simulink model "wlan_phy_rx_pmd"  (Simulink 7.8)
settings: Solver=VariableStepDiscrete  SolverMode=SingleTasking  StartTime=0.0  StopTime=simtime  RelTol=1e-3
PostLoadFcn:
  | wlan_phy_rx_init
InitFcn:
  | wlan_phy_rx_init
StartFcn:
  | wlan_phy_rx_init
values from neighbouring files (symbols this model uses):
  barker_seq20 = 0.95 * circshift(barker_seq20, [0 4]) ./ max(abs(barker_seq20))    [wlan_phy_rx_init.m]
  bit_scrambler_lfsr_bytes = bi2de(reshape(repmat(scr, 1, 8), 8, 127)', 'left-msb')    [wlan_phy_rx_init.m]
  deinterleave_ROM = [deinterleave_ROM interleave_64QAM zeros(1, 512-length(interleave_64QAM))]    [wlan_phy_rx_init.m]
  longCorr_coef_i = [3*real(LTS_corr)]    [wlan_phy_rx_init.m]
  longCorr_coef_q = [3*imag(LTS_corr)]    [wlan_phy_rx_init.m]
  MAX_CP_LEN = 32    [wlan_phy_rx_init.m]
  MAX_NCBPS = 288    [wlan_phy_rx_init.m]
  MAX_NUM_BYTES = 4096    [wlan_phy_rx_init.m]
  MAX_NUM_SC = 64    [wlan_phy_rx_init.m]
  MAX_NUM_SYMS = 600    [wlan_phy_rx_init.m]
  n_bits_preFFT_sampBuff = ceil(log2(4*MAX_NUM_SC))    [wlan_phy_rx_init.m]
  NCBPS_16QAM = 192    [wlan_phy_rx_init.m]
  NCBPS_64QAM = 288    [wlan_phy_rx_init.m]
  NCBPS_BPSK = 48    [wlan_phy_rx_init.m]
  NCBPS_QPSK = 96    [wlan_phy_rx_init.m]
  NDBPS_16QAM12 = 96    [wlan_phy_rx_init.m]
  NDBPS_16QAM34 = 144    [wlan_phy_rx_init.m]
  NDBPS_64QAM23 = 192    [wlan_phy_rx_init.m]
  NDBPS_64QAM34 = 216    [wlan_phy_rx_init.m]
  NDBPS_BPSK12 = 24    [wlan_phy_rx_init.m]
  NDBPS_BPSK34 = 36    [wlan_phy_rx_init.m]
  NDBPS_QPSK12 = 48    [wlan_phy_rx_init.m]
  NDBPS_QPSK34 = 72    [wlan_phy_rx_init.m]
  paylod_vec_samp_time = 8    [wlan_phy_rx_init.m]
  PHY_MIN_PKT_LEN = 14    [wlan_phy_rx_init.m]
  scr_ind_rev = zeros(1,128)    [wlan_phy_rx_init.m]
undefined symbols (not defined in the file or in its folder): gcbh, xlBlockLoadCallback, xlGetNormalizedPeriod, xlipgetpartsetting
libraries: xbsIndex_r4 x3119, simulink x1, xbsEDKLib_r4 x1
(part 4 of 19)

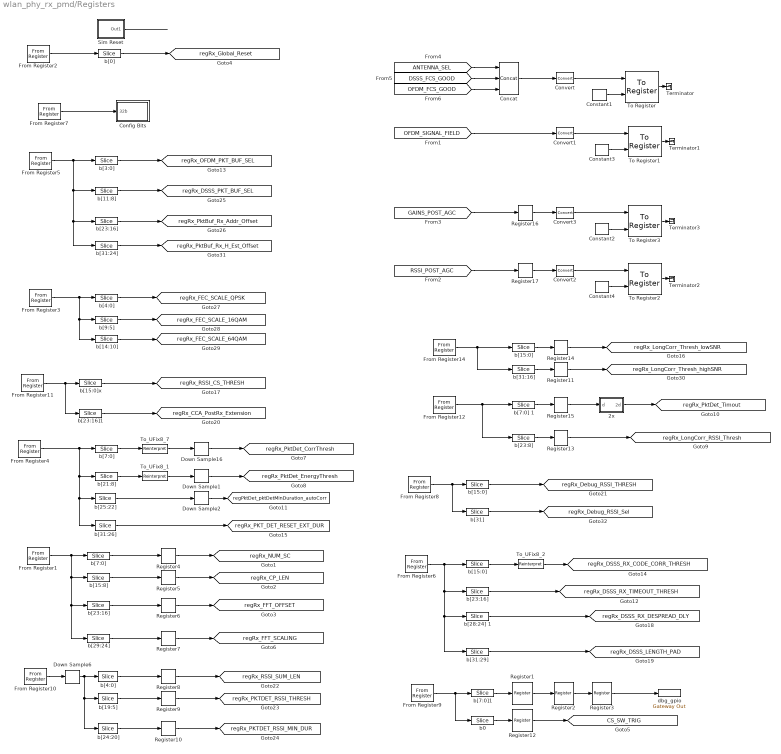
== system wlan_phy_rx_pmd/Registers ==
  " b[23:16] " : xbsIndex_r4/Slice  nbits=8  boolean_output=off  mode=Lower Bit Location + Width  bit1=0  base1=MSB of Input  bit0=16  base0=LSB of Input  dbl_ovrd=off  block_type=slice
      settings: block_version=VER_STRING_GOES_HERE
  " b[31:24]" : xbsIndex_r4/Slice  nbits=8  boolean_output=off  mode=Lower Bit Location + Width  bit1=0  base1=MSB of Input  bit0=24  base0=LSB of Input  dbl_ovrd=off  block_type=slice
      settings: block_version=VER_STRING_GOES_HERE
  "2x " : Subsystem
  Concat : xbsIndex_r4/Concat  num_inputs=3  dbl_ovrd=off  block_type=concat
      settings: block_version=8.2.02
  Config Bits : Subsystem
  Constant{1..4} (4 alike) : xbsIndex_r4/Constant  const=1  gui_display_data_type=Boolean  arith_type=Boolean  n_bits=30  bin_pt=0  preci_type=Single  exp_width=8  frac_width=24  explicit_period=on  period=1  equ=P=C  opselect=C  inp2=PCIN>>17  opr=+  inp1=P  carry=CIN  dbl_ovrd=off  block_type=constant
  Convert{,1..3} (4 alike) : xbsIndex_r4/Convert  gui_display_data_type=Fixed-point  arith_type=Unsigned  n_bits=32  bin_pt=0  float_type=Single  exp_bits=8  fraction_bits=24  quantization=Truncate  overflow=Wrap  en=off  latency=0  dbl_ovrd=off  pipeline=off  block_type=convert
  Down Sample1 : xbsIndex_r4/Down Sample  sample_ratio=8  sample_phase="Last Value of Frame  (most efficient)"  en=off  latency=1  dbl_ovrd=off  block_type=dsamp
      settings: block_version=VER_STRING_GOES_HERE
  Down Sample16 : xbsIndex_r4/Down Sample  sample_ratio=8  sample_phase="Last Value of Frame  (most efficient)"  en=off  latency=1  dbl_ovrd=off  block_type=dsamp
      settings: block_version=VER_STRING_GOES_HERE
  Down Sample2 : xbsIndex_r4/Down Sample  sample_ratio=8  sample_phase="Last Value of Frame  (most efficient)"  en=off  latency=1  dbl_ovrd=off  block_type=dsamp
      settings: block_version=VER_STRING_GOES_HERE
  Down Sample6 : xbsIndex_r4/Down Sample  sample_ratio=16  sample_phase="Last Value of Frame  (most efficient)"  en=off  latency=1  dbl_ovrd=off  block_type=dsamp
      settings: block_version=VER_STRING_GOES_HERE
  From Register1 : xbsIndex_r4/From Register  shared_memory_name='FFT_Config'  init=REG_RX_FFT_Config  period=1  ownership=Locally owned and initialized  gui_display_data_type=Fixed-point  arith_type=Unsigned  n_bits=32  bin_pt=0  preci_type=Single  dbl_ovrd=off  block_type=fromreg
      settings: AttributesFormatString=<< %<shared_memory_name> >>  infoedit="Register block that reads data to a shared memory register.  Delay of one sample period."
  From Register10 : xbsIndex_r4/From Register  shared_memory_name='PKTDET_RSSI_CONFIG'  init=REG_RX_PKTDET_RSSI_CONFIG  period=1  ownership=Locally owned and initialized  gui_display_data_type=Fixed-point  arith_type=Unsigned  n_bits=32  bin_pt=0  preci_type=Single  dbl_ovrd=off  block_type=fromreg
      settings: AttributesFormatString=<< %<shared_memory_name> >>  infoedit="Register block that reads data to a shared memory register.  Delay of one sample period."
  From Register11 : xbsIndex_r4/From Register  shared_memory_name='PHY_CCA_CONFIG'  init=REG_RX_CCA_CONFIG  period=1  ownership=Locally owned and initialized  gui_display_data_type=Fixed-point  arith_type=Unsigned  n_bits=32  bin_pt=0  preci_type=Single  dbl_ovrd=off  block_type=fromreg
      settings: AttributesFormatString=<< %<shared_memory_name> >>  infoedit="Register block that reads data to a shared memory register.  Delay of one sample period."
  From Register12 : xbsIndex_r4/From Register  shared_memory_name='LTS_Corr_Config'  init=REG_RX_LTS_Corr_Confg  period=1  ownership=Locally owned and initialized  gui_display_data_type=Fixed-point  arith_type=Unsigned  n_bits=32  bin_pt=0  preci_type=Single  dbl_ovrd=off  block_type=fromreg
      settings: AttributesFormatString=<< %<shared_memory_name> >>  infoedit="Register block that reads data to a shared memory register.  Delay of one sample period."
  From Register14 : xbsIndex_r4/From Register  shared_memory_name='LTS_Corr_Thresh'  init=REG_RX_LTS_Corr_Thresh  period=1  ownership=Locally owned and initialized  gui_display_data_type=Fixed-point  arith_type=Unsigned  n_bits=32  bin_pt=0  preci_type=Single  dbl_ovrd=off  block_type=fromreg
      settings: AttributesFormatString=<< %<shared_memory_name> >>  infoedit="Register block that reads data to a shared memory register.  Delay of one sample period."
  From Register2 : xbsIndex_r4/From Register  shared_memory_name='Control'  init=REG_RX_Control  period=1  ownership=Locally owned and initialized  gui_display_data_type=Fixed-point  arith_type=Unsigned  n_bits=32  bin_pt=0  preci_type=Single  dbl_ovrd=off  block_type=fromreg
      settings: AttributesFormatString=<< %<shared_memory_name> >>  infoedit="Register block that reads data to a shared memory register.  Delay of one sample period."
  From Register3 : xbsIndex_r4/From Register  shared_memory_name='FEC_CONFIG'  init=REG_RX_FEC_Config  period=1  ownership=Locally owned and initialized  gui_display_data_type=Fixed-point  arith_type=Unsigned  n_bits=32  bin_pt=0  preci_type=Single  dbl_ovrd=off  block_type=fromreg
      settings: AttributesFormatString=<< %<shared_memory_name> >>  infoedit="Register block that reads data to a shared memory register.  Delay of one sample period."
  From Register4 : xbsIndex_r4/From Register  shared_memory_name='PKTDET_AUTOCORR_CONFIG'  init=REG_RX_PktDet_AutoCorr_Config  period=1  ownership=Locally owned and initialized  gui_display_data_type=Fixed-point  arith_type=Unsigned  n_bits=32  bin_pt=0  preci_type=Single  dbl_ovrd=off  block_type=fromreg
      settings: AttributesFormatString=<< %<shared_memory_name> >>  infoedit="Register block that reads data to a shared memory register.  Delay of one sample period."
  From Register5 : xbsIndex_r4/From Register  shared_memory_name='PKT_BUF_SEL'  init=REG_RX_PktBuf_Sel  period=1  ownership=Locally owned and initialized  gui_display_data_type=Fixed-point  arith_type=Unsigned  n_bits=32  bin_pt=0  preci_type=Single  dbl_ovrd=off  block_type=fromreg
      settings: AttributesFormatString=<< %<shared_memory_name> >>  infoedit="Register block that reads data to a shared memory register.  Delay of one sample period."
  From Register6 : xbsIndex_r4/From Register  shared_memory_name='DSSS_RX_CONFIG'  init=REG_RX_DSSS_RX_CONFIG  period=1  ownership=Locally owned and initialized  gui_display_data_type=Fixed-point  arith_type=Unsigned  n_bits=32  bin_pt=0  preci_type=Single  dbl_ovrd=off  block_type=fromreg
      settings: AttributesFormatString=<< %<shared_memory_name> >>  infoedit="Register block that reads data to a shared memory register.  Delay of one sample period."
  From Register7 : xbsIndex_r4/From Register  shared_memory_name='CONFIG'  init=REG_RX_Config  period=1  ownership=Locally owned and initialized  gui_display_data_type=Fixed-point  arith_type=Unsigned  n_bits=32  bin_pt=0  preci_type=Single  dbl_ovrd=off  block_type=fromreg
      settings: AttributesFormatString=<< %<shared_memory_name> >>  infoedit="Register block that reads data to a shared memory register.  Delay of one sample period."
  From Register8 : xbsIndex_r4/From Register  shared_memory_name='RSSI_THRESH'  init=600  period=1  ownership=Locally owned and initialized  gui_display_data_type=Fixed-point  arith_type=Unsigned  n_bits=32  bin_pt=0  preci_type=Single  dbl_ovrd=off  block_type=fromreg
      settings: AttributesFormatString=<< %<shared_memory_name> >>  infoedit="Register block that reads data to a shared memory register.  Delay of one sample period."
  From Register9 : xbsIndex_r4/From Register  shared_memory_name='DEBUG_GPIO'  init=0  period=1  ownership=Locally owned and initialized  gui_display_data_type=Fixed-point  arith_type=Unsigned  n_bits=32  bin_pt=0  preci_type=Single  dbl_ovrd=off  block_type=fromreg
      settings: AttributesFormatString=<< %<shared_memory_name> >>  infoedit="Register block that reads data to a shared memory register.  Delay of one sample period."
  Register{1..17} (17 alike) : xbsIndex_r4/Register  init=0  rst=off  en=off  dbl_ovrd=off  block_type=register
  Sim Reset : Subsystem
  Terminator{,1..3} (4 alike) : Terminator
  To Register : xbsIndex_r4/To Register  shared_memory_name='Status'  init=0  ownership=Locally owned and initialized  explicit_data_type=on  gui_display_data_type=Fixed-point  arith_type=Unsigned  n_bits=32  bin_pt=0  preci_type=Single  dbl_ovrd=off  block_type=toreg
      settings: AttributesFormatString=<< %<shared_memory_name> >>  infoedit="Register block that writes data to a shared memory register.  Delay of one sample period."
  To Register1 : xbsIndex_r4/To Register  shared_memory_name='SIGNAL_FIELD'  init=0  ownership=Locally owned and initialized  explicit_data_type=on  gui_display_data_type=Fixed-point  arith_type=Unsigned  n_bits=32  bin_pt=0  preci_type=Single  dbl_ovrd=off  block_type=toreg
      settings: AttributesFormatString=<< %<shared_memory_name> >>  infoedit="Register block that writes data to a shared memory register.  Delay of one sample period."
  To Register2 : xbsIndex_r4/To Register  shared_memory_name='RX_PKT_RSSI_AB'  init=0  ownership=Locally owned and initialized  explicit_data_type=on  gui_display_data_type=Fixed-point  arith_type=Unsigned  n_bits=32  bin_pt=0  preci_type=Single  dbl_ovrd=off  block_type=toreg
      settings: AttributesFormatString=<< %<shared_memory_name> >>  infoedit="Register block that writes data to a shared memory register.  Delay of one sample period."
  To Register3 : xbsIndex_r4/To Register  shared_memory_name='RX_PKT_AGC_GAINS'  init=0  ownership=Locally owned and initialized  explicit_data_type=on  gui_display_data_type=Fixed-point  arith_type=Unsigned  n_bits=32  bin_pt=0  preci_type=Single  dbl_ovrd=off  block_type=toreg
      settings: AttributesFormatString=<< %<shared_memory_name> >>  infoedit="Register block that writes data to a shared memory register.  Delay of one sample period."
  To_UFix8_1 : xbsIndex_r4/Reinterpret  force_arith_type=on  arith_type=Unsigned  force_bin_pt=on  bin_pt=8  block_type=cast
      settings: block_version=VER_STRING_GOES_HERE
  To_UFix8_2 : xbsIndex_r4/Reinterpret  force_arith_type=on  arith_type=Unsigned  force_bin_pt=on  bin_pt=8  block_type=cast
      settings: block_version=VER_STRING_GOES_HERE
  To_UFix8_7 : xbsIndex_r4/Reinterpret  force_arith_type=on  arith_type=Unsigned  force_bin_pt=on  bin_pt=8  block_type=cast
      settings: block_version=VER_STRING_GOES_HERE
  b0 : xbsIndex_r4/Slice  nbits=1  boolean_output=off  mode=Lower Bit Location + Width  bit1=0  base1=MSB of Input  bit0=0  base0=LSB of Input  dbl_ovrd=off  block_type=slice
      settings: block_version=VER_STRING_GOES_HERE
  b[0] : xbsIndex_r4/Slice  nbits=1  boolean_output=off  mode=Lower Bit Location + Width  bit1=0  base1=MSB of Input  bit0=0  base0=LSB of Input  dbl_ovrd=off  block_type=slice
      settings: block_version=VER_STRING_GOES_HERE
  b[11:8] : xbsIndex_r4/Slice  nbits=4  boolean_output=off  mode=Lower Bit Location + Width  bit1=0  base1=MSB of Input  bit0=8  base0=LSB of Input  dbl_ovrd=off  block_type=slice
      settings: block_version=VER_STRING_GOES_HERE
  b[14:10] : xbsIndex_r4/Slice  nbits=5  boolean_output=off  mode=Lower Bit Location + Width  bit1=0  base1=MSB of Input  bit0=10  base0=LSB of Input  dbl_ovrd=off  block_type=slice
      settings: block_version=VER_STRING_GOES_HERE
  b[15:0] : xbsIndex_r4/Slice  nbits=16  boolean_output=off  mode=Lower Bit Location + Width  bit1=0  base1=MSB of Input  bit0=0  base0=LSB of Input  dbl_ovrd=off  block_type=slice
      settings: block_version=VER_STRING_GOES_HERE
  "b[15:0]   " : xbsIndex_r4/Slice  nbits=16  boolean_output=off  mode=Lower Bit Location + Width  bit1=0  base1=MSB of Input  bit0=0  base0=LSB of Input  dbl_ovrd=off  block_type=slice
      settings: block_version=VER_STRING_GOES_HERE
  "b[15:0]    " : xbsIndex_r4/Slice  nbits=16  boolean_output=off  mode=Lower Bit Location + Width  bit1=0  base1=MSB of Input  bit0=0  base0=LSB of Input  dbl_ovrd=off  block_type=slice
      settings: block_version=VER_STRING_GOES_HERE
  b[15:0]x : xbsIndex_r4/Slice  nbits=16  boolean_output=off  mode=Lower Bit Location + Width  bit1=0  base1=MSB of Input  bit0=0  base0=LSB of Input  dbl_ovrd=off  block_type=slice
      settings: block_version=VER_STRING_GOES_HERE
  b[15:8] : xbsIndex_r4/Slice  nbits=8  boolean_output=off  mode=Lower Bit Location + Width  bit1=0  base1=MSB of Input  bit0=8  base0=LSB of Input  dbl_ovrd=off  block_type=slice
      settings: block_version=VER_STRING_GOES_HERE
  b[19:5] : xbsIndex_r4/Slice  nbits=15  boolean_output=off  mode=Lower Bit Location + Width  bit1=0  base1=MSB of Input  bit0=5  base0=LSB of Input  dbl_ovrd=off  block_type=slice
      settings: block_version=VER_STRING_GOES_HERE
  "b[21:8] " : xbsIndex_r4/Slice  nbits=14  boolean_output=off  mode=Lower Bit Location + Width  bit1=0  base1=MSB of Input  bit0=8  base0=LSB of Input  dbl_ovrd=off  block_type=slice
      settings: block_version=VER_STRING_GOES_HERE
  b[23:16] : xbsIndex_r4/Slice  nbits=8  boolean_output=off  mode=Lower Bit Location + Width  bit1=0  base1=MSB of Input  bit0=16  base0=LSB of Input  dbl_ovrd=off  block_type=slice
      settings: block_version=VER_STRING_GOES_HERE
  "b[23:16]   " : xbsIndex_r4/Slice  nbits=8  boolean_output=off  mode=Lower Bit Location + Width  bit1=0  base1=MSB of Input  bit0=16  base0=LSB of Input  dbl_ovrd=off  block_type=slice
      settings: block_version=VER_STRING_GOES_HERE
  b[23:16]1 : xbsIndex_r4/Slice  nbits=8  boolean_output=off  mode=Lower Bit Location + Width  bit1=0  base1=MSB of Input  bit0=16  base0=LSB of Input  dbl_ovrd=off  block_type=slice
      settings: block_version=VER_STRING_GOES_HERE
  b[23:8] : xbsIndex_r4/Slice  nbits=16  boolean_output=off  mode=Lower Bit Location + Width  bit1=0  base1=MSB of Input  bit0=8  base0=LSB of Input  dbl_ovrd=off  block_type=slice
      settings: block_version=VER_STRING_GOES_HERE
  b[24:20] : xbsIndex_r4/Slice  nbits=5  boolean_output=off  mode=Lower Bit Location + Width  bit1=0  base1=MSB of Input  bit0=20  base0=LSB of Input  dbl_ovrd=off  block_type=slice
      settings: block_version=VER_STRING_GOES_HERE
  "b[25:22] " : xbsIndex_r4/Slice  nbits=4  boolean_output=off  mode=Lower Bit Location + Width  bit1=0  base1=MSB of Input  bit0=22  base0=LSB of Input  dbl_ovrd=off  block_type=slice
      settings: block_version=VER_STRING_GOES_HERE
  b[28:24] 1 : xbsIndex_r4/Slice  nbits=5  boolean_output=off  mode=Lower Bit Location + Width  bit1=0  base1=MSB of Input  bit0=24  base0=LSB of Input  dbl_ovrd=off  block_type=slice
      settings: block_version=VER_STRING_GOES_HERE
  b[29:24] : xbsIndex_r4/Slice  nbits=6  boolean_output=off  mode=Lower Bit Location + Width  bit1=0  base1=MSB of Input  bit0=24  base0=LSB of Input  dbl_ovrd=off  block_type=slice
      settings: block_version=VER_STRING_GOES_HERE
  b[31:16] : xbsIndex_r4/Slice  nbits=16  boolean_output=off  mode=Lower Bit Location + Width  bit1=0  base1=MSB of Input  bit0=16  base0=LSB of Input  dbl_ovrd=off  block_type=slice
      settings: block_version=VER_STRING_GOES_HERE
  b[31:26] : xbsIndex_r4/Slice  nbits=6  boolean_output=off  mode=Lower Bit Location + Width  bit1=0  base1=MSB of Input  bit0=26  base0=LSB of Input  dbl_ovrd=off  block_type=slice
      settings: block_version=VER_STRING_GOES_HERE
  b[31:29] : xbsIndex_r4/Slice  nbits=3  boolean_output=off  mode=Lower Bit Location + Width  bit1=0  base1=MSB of Input  bit0=29  base0=LSB of Input  dbl_ovrd=off  block_type=slice
      settings: block_version=VER_STRING_GOES_HERE
  b[31] : xbsIndex_r4/Slice  nbits=1  boolean_output=off  mode=Lower Bit Location + Width  bit1=0  base1=MSB of Input  bit0=31  base0=LSB of Input  dbl_ovrd=off  block_type=slice
      settings: block_version=VER_STRING_GOES_HERE
  b[3:0] : xbsIndex_r4/Slice  nbits=4  boolean_output=off  mode=Lower Bit Location + Width  bit1=0  base1=MSB of Input  bit0=0  base0=LSB of Input  dbl_ovrd=off  block_type=slice
      settings: block_version=VER_STRING_GOES_HERE
  b[4:0] : xbsIndex_r4/Slice  nbits=5  boolean_output=off  mode=Lower Bit Location + Width  bit1=0  base1=MSB of Input  bit0=0  base0=LSB of Input  dbl_ovrd=off  block_type=slice
      settings: block_version=VER_STRING_GOES_HERE
  "b[4:0]  " : xbsIndex_r4/Slice  nbits=5  boolean_output=off  mode=Lower Bit Location + Width  bit1=0  base1=MSB of Input  bit0=0  base0=LSB of Input  dbl_ovrd=off  block_type=slice
      settings: block_version=VER_STRING_GOES_HERE
  b[7:0] : xbsIndex_r4/Slice  nbits=8  boolean_output=off  mode=Lower Bit Location + Width  bit1=0  base1=MSB of Input  bit0=0  base0=LSB of Input  dbl_ovrd=off  block_type=slice
      settings: block_version=VER_STRING_GOES_HERE
  "b[7:0] " : xbsIndex_r4/Slice  nbits=8  boolean_output=off  mode=Lower Bit Location + Width  bit1=0  base1=MSB of Input  bit0=0  base0=LSB of Input  dbl_ovrd=off  block_type=slice
      settings: block_version=VER_STRING_GOES_HERE
  b[7:0] 1 : xbsIndex_r4/Slice  nbits=8  boolean_output=off  mode=Lower Bit Location + Width  bit1=0  base1=MSB of Input  bit0=0  base0=LSB of Input  dbl_ovrd=off  block_type=slice
      settings: block_version=VER_STRING_GOES_HERE
  b[7:0]1 : xbsIndex_r4/Slice  nbits=8  boolean_output=off  mode=Lower Bit Location + Width  bit1=0  base1=MSB of Input  bit0=0  base0=LSB of Input  dbl_ovrd=off  block_type=slice
      settings: block_version=VER_STRING_GOES_HERE
  b[9:5] : xbsIndex_r4/Slice  nbits=5  boolean_output=off  mode=Lower Bit Location + Width  bit1=0  base1=MSB of Input  bit0=5  base0=LSB of Input  dbl_ovrd=off  block_type=slice
      settings: block_version=VER_STRING_GOES_HERE
  dbg_gpio : xbsIndex_r4/Gateway Out  inherit_from_input=off  hdl_port=on  timing_constraint=None  locs_specified=off  LOCs={}  UseAsDAC=off  DACChannel='1'  block_type=gatewayout
  tag scope: CS_SW_TRIG=global, regPktDet_pktDetMinDuration_autoCorr=global, regRx_CCA_PostRx_Extension=global, regRx_CP_LEN=global, regRx_DSSS_LENGTH_PAD=global, regRx_DSSS_PKT_BUF_SEL=global, regRx_DSSS_RX_CODE_CORR_THRESH=global, regRx_DSSS_RX_DESPREAD_DLY=global, regRx_DSSS_RX_TIMEOUT_THRESH=global, regRx_Debug_RSSI_Sel=global, regRx_Debug_RSSI_THRESH=global, regRx_FEC_SCALE_16QAM=global, regRx_FEC_SCALE_64QAM=global, regRx_FEC_SCALE_QPSK=global, regRx_FFT_OFFSET=global, regRx_FFT_SCALING=global, regRx_Global_Reset=global, regRx_LongCorr_RSSI_Thresh=global, regRx_LongCorr_Thresh_highSNR=global, regRx_LongCorr_Thresh_lowSNR=global, regRx_NUM_SC=global, regRx_OFDM_PKT_BUF_SEL=global, regRx_PKTDET_RSSI_MIN_DUR=global, regRx_PKTDET_RSSI_THRESH=global, regRx_PKT_DET_RESET_EXT_DUR=global, regRx_PktBuf_Rx_Addr_Offset=global, regRx_PktBuf_Rx_H_Est_Offset=global, regRx_PktDet_CorrThresh=global, regRx_PktDet_EnergyThresh=global, regRx_PktDet_Timout=global, regRx_RSSI_CS_THRESH=global, regRx_RSSI_SUM_LEN=global
  wires:
  b[31:16]/1 -> Register11/1
  From Register1/1 -> b[7:0]/1, b[15:8]/1, b[23:16]/1, b[29:24]/1
  b[7:0]/1 -> Register4/1
  b[15:8]/1 -> Register5/1
  b[23:16]/1 -> Register6/1
  From Register2/1 -> b[0]/1
  b[0]/1 -> [regRx_Global_Reset]
  From Register3/1 -> "b[4:0]  "/1, b[9:5]/1, b[14:10]/1
  b[29:24]/1 -> Register7/1
  [DSSS_FCS_GOOD] -> Concat/2
  [OFDM_FCS_GOOD] -> Concat/3
  Constant1/1 -> To Register/2
  To Register/1 -> Terminator/1
  From Register4/1 -> "b[7:0] "/1, "b[21:8] "/1, "b[25:22] "/1, b[31:26]/1
  "b[7:0] "/1 -> To_UFix8_7/1
  "b[21:8] "/1 -> To_UFix8_1/1
  To_UFix8_7/1 -> Down Sample16/1
  To_UFix8_1/1 -> Down Sample1/1
  Down Sample16/1 -> [regRx_PktDet_CorrThresh]
  Down Sample1/1 -> [regRx_PktDet_EnergyThresh]
  b[15:0]/1 -> Register14/1
  "b[25:22] "/1 -> Down Sample2/1
  Down Sample2/1 -> [regPktDet_pktDetMinDuration_autoCorr]
  Sim Reset/1(Out1) -> ?
  From Register5/1 -> b[3:0]/1, b[11:8]/1, " b[23:16] "/1, " b[31:24]"/1
  b[3:0]/1 -> [regRx_OFDM_PKT_BUF_SEL]
  From Register6/1 -> "b[15:0]   "/1, "b[23:16]   "/1, b[28:24] 1/1, b[31:29]/1
  "b[15:0]   "/1 -> To_UFix8_2/1
  "b[23:16]   "/1 -> [regRx_DSSS_RX_TIMEOUT_THRESH]
  To_UFix8_2/1 -> [regRx_DSSS_RX_CODE_CORR_THRESH]
  Concat/1 -> Convert/1
  b[28:24] 1/1 -> [regRx_DSSS_RX_DESPREAD_DLY]
  b[31:29]/1 -> [regRx_DSSS_LENGTH_PAD]
  From Register8/1 -> "b[15:0]    "/1, b[31]/1
  "b[15:0]    "/1 -> [regRx_Debug_RSSI_THRESH]
  From Register9/1 -> b[7:0]1/1, b0/1
  b[7:0]1/1 -> Register1/1
  Register1/1 -> Register2/1
  Register2/1 -> Register3/1
  Register3/1 -> dbg_gpio/1
  b[4:0]/1 -> Register8/1
  Down Sample6/1 -> b[4:0]/1, b[19:5]/1, b[24:20]/1
  b[19:5]/1 -> Register9/1
  From Register10/1 -> Down Sample6/1
  b[24:20]/1 -> Register10/1
  From Register7/1 -> Config Bits/1(32b)
  Register4/1 -> [regRx_NUM_SC]
  Register5/1 -> [regRx_CP_LEN]
  Register6/1 -> [regRx_FFT_OFFSET]
  Register7/1 -> [regRx_FFT_SCALING]
  Register8/1 -> [regRx_RSSI_SUM_LEN]
  Register9/1 -> [regRx_PKTDET_RSSI_THRESH]
  Register10/1 -> [regRx_PKTDET_RSSI_MIN_DUR]
  Register11/1 -> [regRx_LongCorr_Thresh_highSNR]
  b[31:26]/1 -> [regRx_PKT_DET_RESET_EXT_DUR]
  From Register11/1 -> b[15:0]x/1, b[23:16]1/1
  b[15:0]x/1 -> [regRx_RSSI_CS_THRESH]
  b[23:16]1/1 -> [regRx_CCA_PostRx_Extension]
  b[11:8]/1 -> [regRx_DSSS_PKT_BUF_SEL]
  Convert/1 -> To Register/1
  " b[23:16] "/1 -> [regRx_PktBuf_Rx_Addr_Offset]
  "b[4:0]  "/1 -> [regRx_FEC_SCALE_QPSK]
  b[9:5]/1 -> [regRx_FEC_SCALE_16QAM]
  b[14:10]/1 -> [regRx_FEC_SCALE_64QAM]
  Constant3/1 -> To Register1/2
  To Register1/1 -> Terminator1/1
  [OFDM_SIGNAL_FIELD] -> Convert1/1
  Convert1/1 -> To Register1/1
  b0/1 -> Register12/1
  Register12/1 -> [CS_SW_TRIG]
  Constant4/1 -> To Register2/2
  To Register2/1 -> Terminator2/1
  Convert2/1 -> To Register2/1
  Register13/1 -> [regRx_LongCorr_RSSI_Thresh]
  b[23:8]/1 -> Register13/1
  Register14/1 -> [regRx_LongCorr_Thresh_lowSNR]
  From Register14/1 -> b[15:0]/1, b[31:16]/1
  From Register12/1 -> b[7:0] 1/1, b[23:8]/1
  "2x "/1(2d) -> [regRx_PktDet_Timout]
  Register15/1 -> "2x "/1(d)
  b[7:0] 1/1 -> Register15/1
  " b[31:24]"/1 -> [regRx_PktBuf_Rx_H_Est_Offset]
  [RSSI_POST_AGC] -> Register17/1
  [ANTENNA_SEL] -> Concat/1
  Constant2/1 -> To Register3/2
  To Register3/1 -> Terminator3/1
  Convert3/1 -> To Register3/1
  [GAINS_POST_AGC] -> Register16/1
  Register16/1 -> Convert3/1
  Register17/1 -> Convert2/1
  b[31]/1 -> [regRx_Debug_RSSI_Sel]

== system wlan_phy_rx_pmd/Resets ==
  Convert1 : xbsIndex_r4/Convert  gui_display_data_type=Boolean  arith_type=Boolean  n_bits=16  bin_pt=14  float_type=Single  exp_bits=8  fraction_bits=24  quantization=Truncate  overflow=Wrap  en=off  latency=0  dbl_ovrd=off  pipeline=off  block_type=convert
  Pulse Generator : DiscretePulseGenerator  Period=1e9  PulseWidth=8  PhaseDelay=100
  tag scope: RX_GLOBAL_RESET=global
  wires:
  [regRx_Global_Reset] -> Convert1/1
  Convert1/1 -> [RX_GLOBAL_RESET]

== system wlan_phy_rx_pmd/Sync & Antenna Sel ==
  Rx I : Inport
  Rx Q : Inport  Port=2
  IQ Valid : Inport  Port=3
  RSSI : Inport  Port=4
  Reset : Inport  Port=5
  ADC/Corr : Scope  NumInputPorts=10
      settings: TimeRange=16708  YMin=0~-1~-1~0~-1~-1~-1~-1~-1~-1  YMax=1~1~1~1~1~1~1~1~1~1  SaveName=ScopeData34
  Antenna Selection : Subsystem
  Debug  Outputs : Subsystem
  From Workspace1 : FromWorkspace  VariableName=samps_pkt_det  SampleTime=paylod_vec_samp_time  Interpolate=off  OutputAfterFinalValue=Setting to zero
  Gateway Out{,1..3} (4 alike) : xbsIndex_r4/Gateway Out  inherit_from_input=off  hdl_port=off  timing_constraint=None  locs_specified=off  LOCs={}  UseAsDAC=off  DACChannel='1'  block_type=gatewayout
  LTS Correlation : Subsystem
  PHY CCA : Subsystem
  Pkt Det : Subsystem
  Pkt Det AGC Ctrl : Subsystem
  Resets : Subsystem
  " Rx I" : Outport
  " Rx Q" : Outport  Port=2
  " IQ Valid" : Outport  Port=3
  " Pkt Det" : Outport  Port=4
  LTS Sync : Outport  Port=5
  wires:
  LTS Correlation/1(Det) -> LTS Sync/1, PHY CCA/1(LTS Sync), Debug  Outputs/2(LTS)
  LTS Correlation/2(Timeout) -> PHY CCA/2(LTS Timeout), Resets/3(LTS Timeout), Debug  Outputs/3(LTS Timeout), Gateway Out2/1
  From Workspace1/1 -> ADC/Corr/10  "Capt Pkt Det"
  Gateway Out2/1 -> ADC/Corr/3  "LTS TO"
  Gateway Out1/1 -> ADC/Corr/2  "Pkt Done"
  Gateway Out/1 -> ADC/Corr/1  "Pkt Det"
  Pkt Det/1(Pkt Det) -> Antenna Selection/1(Pkt Det), LTS Correlation/5(En), PHY CCA/4(Pkt Det), Debug  Outputs/1(Pkt Det), " Pkt Det"/1, Pkt Det AGC Ctrl/1(Pkt Det), Resets/1(Det), Gateway Out/1
  Resets/1(Reset) -> Pkt Det/1(Reset), Antenna Selection/6(Reset), LTS Correlation/6(Reset), PHY CCA/6(Reset), Gateway Out1/1
  Reset/1 -> Resets/2(OFDM Reset)
  IQ Valid/1 -> Pkt Det/4(IQ Valid), Antenna Selection/4(IQ Valid)
  RSSI/1 -> Pkt Det/5(RSSI Vec), Antenna Selection/5(RSSI Vec), PHY CCA/5(Avg RSSI Vec)
  Rx I/1 -> Pkt Det/2(Rx I Vec), Antenna Selection/2(Rx I Vec)
  Rx Q/1 -> Pkt Det/3(Rx Q Vec), Antenna Selection/3(Rx Q Vec)
  Antenna Selection/1(Rx I) -> " Rx I"/1, LTS Correlation/1(rx_I)
  Antenna Selection/2(" Rx Q") -> " Rx Q"/1, LTS Correlation/2(rx_Q)
  Antenna Selection/3(" IQ Valid") -> " IQ Valid"/1, LTS Correlation/3(rx_IQ_valid), PHY CCA/3(IQ Valid)
  Antenna Selection/4(RSSI) -> LTS Correlation/4(RSSI), Gateway Out3/1  "Avg RSSI"
  Gateway Out3/1 -> ADC/Corr/9

== system wlan_phy_rx_pmd/CFO & Samp Buffer/CFO Correction ==
  note: DDS requires >1 cycle EN early to get setup
      | Use gloabl reset for this
      | Safe, since DDS output is ignored when
      | it's not being used, so bogus outputs
      | between packets is no problem
      |
      | DDS updates once per input sample
      | Since the FFT only consumes 64 samples
      | per OFDM symbol (ignoring the CP),
      | the DDS keeps running after each FFT
      | frame is loaded. This is fine, since the FFT is
      | configured for non-streaming (it ignores its
      | I/Q inputs while computing a transform)
  "I " : Inport
  "Q " : Inport  Port=2
  DDS Run : Inport  Port=3
  Valid : Inport  Port=4
  tlast : Inport  Port=5
  CFO Est : Inport  Port=6
  CFO Corr : Scope  NumInputPorts=8
      settings: TimeRange=35000  YMin=0~-0.4~0~-1~0~0~-0.4~0  YMax=1~0.4~1~2~1~1~0.4~1  SaveName=ScopeData3
  "Complex Multiplier 5.0 " : xbsIndex_r4/Complex Multiplier 5.0   hasatlast=off  hasatuser=on  atuserwidth=1  hasbtlast=off  hasbtuser=off  btuserwidth=1  multtype=Use_Mults  optimizegoal=Performance  flowcontrol=NonBlocking  outputwidth=16  roundmode=Truncate  hasctrltlast=off  hasctrltuser=off  ctrltuserwidth=1  outtlastbehv=Null  latencyconfig=Manual  minimumlatency=2  aclken=off  aresetn=off  trim_axipin_name=on  aportwidth=63  bportwidth=63  ip_name=Complex Multiplier  ip_version=5.0  dsptool_ready=true  ipcore_usecache=true  ipcore_useipmodelcache=true  ipcore_verbose=false  ipcore_latency_parameter='minimumlatency'  wrapper_available=true  port_translation_map={ 'aclken' => 'en', 'aresetn' => 'rst'}  structural_sim=false  block_type=cmpy_v5_0
  Constant1 : xbsIndex_r4/Constant  const=1  gui_display_data_type=Boolean  arith_type=Boolean  n_bits=16  bin_pt=14  preci_type=Single  exp_width=8  frac_width=24  explicit_period=off  period=8  equ=P=C  opselect=C  inp2=PCIN>>17  opr=+  inp1=P  carry=CIN  dbl_ovrd=off  block_type=constant
  Constant2 : xbsIndex_r4/Constant  const=1  gui_display_data_type=Fixed-point  arith_type=Signed (2's comp)  n_bits=16  bin_pt=15  preci_type=Single  exp_width=8  frac_width=24  explicit_period=on  period=1  equ=P=C  opselect=C  inp2=PCIN>>17  opr=+  inp1=P  carry=CIN  dbl_ovrd=off  block_type=constant
  Constant3 : xbsIndex_r4/Constant  const=0  gui_display_data_type=Fixed-point  arith_type=Signed (2's comp)  n_bits=16  bin_pt=15  preci_type=Single  exp_width=8  frac_width=24  explicit_period=on  period=1  equ=P=C  opselect=C  inp2=PCIN>>17  opr=+  inp1=P  carry=CIN  dbl_ovrd=off  block_type=constant
  Constant5 : xbsIndex_r4/Constant  const=1  gui_display_data_type=Boolean  arith_type=Boolean  n_bits=16  bin_pt=14  preci_type=Single  exp_width=8  frac_width=24  explicit_period=off  period=8  equ=P=C  opselect=C  inp2=PCIN>>17  opr=+  inp1=P  carry=CIN  dbl_ovrd=off  block_type=constant
  Convert1 : xbsIndex_r4/Convert  gui_display_data_type=Fixed-point  arith_type="Signed  (2's comp)"  n_bits=16  bin_pt=15  float_type=Single  exp_bits=8  fraction_bits=24  quantization=Truncate  overflow=Wrap  en=off  latency=0  dbl_ovrd=off  pipeline=off  block_type=convert
  Convert2 : xbsIndex_r4/Convert  gui_display_data_type=Fixed-point  arith_type="Signed  (2's comp)"  n_bits=16  bin_pt=15  float_type=Single  exp_bits=8  fraction_bits=24  quantization=Truncate  overflow=Wrap  en=off  latency=0  dbl_ovrd=off  pipeline=off  block_type=convert
  Convert3 : xbsIndex_r4/Convert  gui_display_data_type=Fixed-point  arith_type="Signed  (2's comp)"  n_bits=26  bin_pt=26  float_type=Single  exp_bits=8  fraction_bits=24  quantization=Truncate  overflow=Flag as error  en=off  latency=0  dbl_ovrd=off  pipeline=off  block_type=convert
  Convert4 : xbsIndex_r4/Convert  gui_display_data_type=Fixed-point  arith_type=Unsigned  n_bits=1  bin_pt=0  float_type=Single  exp_bits=8  fraction_bits=24  quantization=Truncate  overflow=Flag as error  en=off  latency=0  dbl_ovrd=off  pipeline=off  block_type=convert
  Convert5 : xbsIndex_r4/Convert  gui_display_data_type=Boolean  arith_type=Boolean  n_bits=1  bin_pt=0  float_type=Single  exp_bits=8  fraction_bits=24  quantization=Truncate  overflow=Flag as error  en=off  latency=0  dbl_ovrd=off  pipeline=off  block_type=convert
  DDS Compiler 4.0 : xbsIndex_r4/DDS Compiler 4.0   partspresent=Phase_Generator_and_SIN_COS_LUT  dds_clock_rate=160  channels=1  parameter_entry=Hardware_Parameters  spurious_free_dynamic_range=36  frequency_resolution=0.4  noise_shaping=None  phase_width=26  output_width=16  output_selection=Sine_and_Cosine  negative_sine=off  negative_cosine=off  amplitude_mode=Full_Range  memory_type=Auto  optimization_goal=Auto  dsp48_use=Minimal  latency_configuration=Auto  latency=6  has_phase_out=off  sclr_pin=off  clock_enable=on  rfd=off  rdy=on  channel_pin=off  explicit_period=off  period=1  phase_increment=Programmable  output_frequency1=0  output_frequency2=0  output_frequency3=0  output_frequency4=0  output_frequency5=0  output_frequency6=0  output_frequency7=0  output_frequency8=0  output_frequency9=0  output_frequency10=0  output_frequency11=0  output_frequency12=0  output_frequency13=0  output_frequency14=0  output_frequency15=0  output_frequency16=0  pinc1='0'  pinc2='0'  pinc3='0'  pinc4='0'  pinc5='0'  pinc6='0'  pinc7='0'  pinc8='0'  pinc9='0'  pinc10='0'  pinc11='0'  pinc12='0'  pinc13='0'  pinc14='0'  pinc15='0'  pinc16='0'  phase_offset=None  phase_offset_angles1=0  phase_offset_angles2=0  phase_offset_angles3=0  phase_offset_angles4=0  phase_offset_angles5=0  phase_offset_angles6=0  phase_offset_angles7=0  phase_offset_angles8=0  phase_offset_angles9=0  phase_offset_angles10=0  phase_offset_angles11=0  phase_offset_angles12=0  phase_offset_angles13=0  phase_offset_angles14=0  phase_offset_angles15=0  phase_offset_angles16=0  poff1='0'  poff2='0'  poff3='0'  poff4='0'  poff5='0'  poff6='0'  poff7='0'  poff8='0'  poff9='0'  poff10='0'  poff11='0'  poff12='0'  poff13='0'  poff14='0'  poff15='0'  poff16='0'  por_mode=false  gui_behaviour=Sysgen  ip_name=DDS Compiler  ip_version=4.0  dsptool_ready=true  wrapper_available=true  port_translation_map={ 'ce' => 'en', 'sclr' => 'rst' }  ipcore_xco_need_fpga_part=true  ipcore_fpga_part=xlipgetpartsetting(gcb, {'virtex4', 'xc4vsx35', '-10', 'ff668'})  ipcore_usecache=true  ipcore_useipmodelcache=true  ipcore_verbose=false  block_type=dds_compiler_v4_0
  Gateway Out{1..7,17} (8 alike) : xbsIndex_r4/Gateway Out  inherit_from_input=off  hdl_port=off  timing_constraint=None  locs_specified=off  LOCs={}  UseAsDAC=off  DACChannel='1'  block_type=gatewayout
  Logical2 : xbsIndex_r4/Logical  logical_function=OR  inputs=3  en=off  latency=0  precision=Full  arith_type=Unsigned  n_bits=16  bin_pt=0  align_bp=on  dbl_ovrd=off  block_type=logical
  Sim Reset : Subsystem
  valid : Outport
  I : Outport  Port=2
  Q : Outport  Port=3
  " tlast" : Outport  Port=4
  wires:
  Constant1/1 -> DDS Compiler 4.0/1
  CFO Est/1 -> Convert3/1
  DDS Run/1 -> Logical2/1
  "Complex Multiplier 5.0 "/2 -> Convert2/1, Gateway Out6/1
  "Complex Multiplier 5.0 "/3 -> Convert1/1
  "I "/1 -> "Complex Multiplier 5.0 "/3, Gateway Out1/1
  "Q "/1 -> "Complex Multiplier 5.0 "/2
  Gateway Out17/1 -> CFO Corr/1
  Gateway Out1/1 -> CFO Corr/2
  Gateway Out2/1 -> CFO Corr/3
  Gateway Out3/1 -> CFO Corr/4
  Gateway Out4/1 -> CFO Corr/5
  DDS Compiler 4.0/2 -> "Complex Multiplier 5.0 "/6, Gateway Out3/1
  Valid/1 -> "Complex Multiplier 5.0 "/1, Gateway Out2/1
  Convert1/1 -> I/1
  Convert2/1 -> Q/1
  "Complex Multiplier 5.0 "/1 -> valid/1, Gateway Out5/1
  DDS Compiler 4.0/3 -> "Complex Multiplier 5.0 "/7
  Logical2/1 -> DDS Compiler 4.0/3, Gateway Out17/1
  [RX_GLOBAL_RESET] -> Logical2/2
  Convert3/1 -> DDS Compiler 4.0/2
  Constant5/1 -> "Complex Multiplier 5.0 "/5
  tlast/1 -> Convert4/1
  "Complex Multiplier 5.0 "/4 -> Gateway Out7/1, Convert5/1
  Gateway Out5/1 -> CFO Corr/6
  Gateway Out6/1 -> CFO Corr/7
  Gateway Out7/1 -> CFO Corr/8
  Convert4/1 -> "Complex Multiplier 5.0 "/4, Gateway Out4/1
  Convert5/1 -> " tlast"/1
  Sim Reset/1(Out1) -> Logical2/3

== system wlan_phy_rx_pmd/CFO & Samp Buffer/CFO Estimation ==
  note: (1/2) for normalization of phase
      | (1/64) for number of summed elements
  LTS_Sync : Inport
  rx_I : Inport  Port=2
  rx_Q : Inport  Port=3
  rx_valid : Inport  Port=4
  CFO Est : Scope  NumInputPorts=10
      settings: TimeRange=10000  YMin=-0.25~-0.25~-0.04~-0.05~-0.3~-0.125~-1~0~-0.000175~-1  YMax=0.3~0.3~0.05~0.04~0.9~0.15~1~1~0~1
  Conj Mult : Subsystem
  Convert2 : xbsIndex_r4/Convert  gui_display_data_type=Fixed-point  arith_type="Signed  (2's comp)"  n_bits=21  bin_pt=13  float_type=Single  exp_bits=8  fraction_bits=24  quantization=Truncate  overflow=Flag as error  en=off  latency=0  dbl_ovrd=off  pipeline=off  block_type=convert
  Delay : xbsIndex_r4/Delay  rst=off  infoeditControl=Selection of Reset will increase slice count due to use of real FFs and instead of SRLs  en=off  latency=8  dbl_ovrd=off  reg_retiming=off  block_type=delay
  Delay1 : xbsIndex_r4/Delay  rst=off  infoeditControl=Selection of Reset will increase slice count due to use of real FFs and instead of SRLs  en=on  latency=64  dbl_ovrd=off  reg_retiming=off  block_type=delay
      settings: xl_area=[7 14 0 14 0 0 0]  block_version=VER_STRING_GOES_HERE
  Delay2 : xbsIndex_r4/Delay  rst=off  infoeditControl=Selection of Reset will increase slice count due to use of real FFs and instead of SRLs  en=on  latency=64  dbl_ovrd=off  reg_retiming=off  block_type=delay
      settings: xl_area=[7 14 0 14 0 0 0]  block_version=VER_STRING_GOES_HERE
  Delay3 : xbsIndex_r4/Delay  rst=off  infoeditControl=Selection of Reset will increase slice count due to use of real FFs and instead of SRLs  en=on  latency=60  dbl_ovrd=off  reg_retiming=off  block_type=delay
      settings: xl_area=[7 14 0 14 0 0 0]  block_version=VER_STRING_GOES_HERE
  Gateway Out{,1..8} (9 alike) : xbsIndex_r4/Gateway Out  inherit_from_input=off  hdl_port=off  timing_constraint=None  locs_specified=off  LOCs={}  UseAsDAC=off  DACChannel='1'  block_type=gatewayout
  Phase : Subsystem
  Posedge3 : Subsystem
  Register : xbsIndex_r4/Register  init=0  rst=on  en=on  dbl_ovrd=off  block_type=register
  Running Sum : Subsystem
  Running Sum1 : Subsystem
  S-R Latch : Subsystem
  Scale : xbsIndex_r4/Scale  scale_factor=-7  dbl_ovrd=off  block_type=scale
      settings: block_version=10.1.2
  CFO : Outport
  wires:
  Gateway Out5/1 -> CFO Est/6  "Sum Q"
  Gateway Out4/1 -> CFO Est/5  "Sum I"
  Gateway Out3/1 -> CFO Est/4  "Conj Prod Q"
  Gateway Out2/1 -> CFO Est/3  "Conj Prod I"
  Gateway Out1/1 -> CFO Est/2  "ADC Q"
  Gateway Out/1 -> CFO Est/1  "ADC I"
  rx_valid/1 -> Conj Mult/1(Valid), Delay2/2, Delay1/2, Delay3/2
  rx_I/1 -> Conj Mult/2(A I), Delay2/1, Gateway Out/1
  rx_Q/1 -> Conj Mult/3(A Q), Delay1/1, Gateway Out1/1
  Delay2/1 -> Conj Mult/4(B I)
  Delay1/1 -> Conj Mult/5(B Q)
  Conj Mult/2(" I") -> Running Sum/2(D), Gateway Out2/1
  Conj Mult/1(" Valid") -> Running Sum/1(En), Running Sum1/1(En)
  Conj Mult/3(" Q") -> Running Sum1/2(D), Gateway Out3/1
  Running Sum/1(Valid) -> Phase/1(Valid)
  Phase/2(Phase) -> Gateway Out6/1, Register/1
  Gateway Out6/1 -> CFO Est/7  "Phase(Sum)"
  Register/1 -> Convert2/1
  Convert2/1 -> Scale/1
  Scale/1 -> CFO/1, Gateway Out8/1
  Gateway Out7/1 -> CFO Est/8  "LTS Sync"
  LTS_Sync/1 -> S-R Latch/1(S)
  Gateway Out8/1 -> CFO Est/9  "CFO Est Out"
  ? -> CFO Est/10
  Delay3/1 -> Posedge3/1(D)
  S-R Latch/1(Q) -> Delay/1, Delay3/1
  Delay/1 -> S-R Latch/2(R)
  Posedge3/1(Q) -> Gateway Out7/1, Register/3
  Running Sum/2(Sum) -> Phase/2(Re), Gateway Out4/1
  Running Sum1/2(Sum) -> Phase/3(Im), Gateway Out5/1
  [regRx_BYPASS_CFO_EST] -> Register/2

== system wlan_phy_rx_pmd/CFO & Samp Buffer/FIFO ==
  note: AND not strictly necessary, since FFT
      | only honors tlast when tvalid is asserted.
      | Explicit AND here makes scopes
      | much easier to understand.
  note: This FIFO aids in synchronizing sample generation
      | and feeding the FFT. It would be possible to design
      | the sample buffer control logic not to require
      | this FIFO, but then the logic would need tweaking
      | if the FFT timing or sample processing timing
      | ever change. This FIFO isolates those blocks,
      | at the cost of a little memory and 1 clock cyle latency
      | (assuming the FFT consumes samples faster than
      | they're received)
  data tvalid : Inport
  I : Inport  Port=2
  Q : Inport  Port=3
  data tlast : Inport  Port=4
  FFT tready : Inport  Port=5
  reset : Inport  Port=6
  AXI FIFO : xbsIndex_r4/AXI FIFO  input_depth_axis=128  actual_fifo_depth_label=130  enable_tdata=on  enable_tdest=off  enable_tstrb=off  enable_tready=on  enable_tid=off  enable_tuser=off  enable_tkeep=off  enable_tlast=on  has_aresetn=off  enable_data_counts_axis=on  fifo_implementation_type_axis=Common Clock Block RAM  trim_axipin_name=on  programmable_full_type_axis=Full  full_threshold_assert_value_axis=0  programmable_empty_type_axis=Empty  empty_threshold_assert_value_axis=1022  block_type=axi_fifo
  FIFO : Scope  NumInputPorts=9
      settings: TimeRange=14580  YMin=0.95~-1~-1~-1~0.95~-1~-1~-1~-1  YMax=1.05~1~1~1~1.05~1~1~1~1  SaveName=ScopeData20
  Gateway Out{1..6,8..9} (8 alike) : xbsIndex_r4/Gateway Out  inherit_from_input=off  hdl_port=off  timing_constraint=None  locs_specified=off  LOCs={}  UseAsDAC=off  DACChannel='1'  block_type=gatewayout
  I/Q Concat : Subsystem
  I/Q Slice : Subsystem
  Inverter : xbsIndex_r4/Inverter  en=off  latency=0  dbl_ovrd=off  block_type=inv
  Logical2 : xbsIndex_r4/Logical  logical_function=AND  inputs=2  en=off  latency=0  precision=Full  arith_type=Unsigned  n_bits=16  bin_pt=0  align_bp=on  dbl_ovrd=off  block_type=logical
  Register2 : xbsIndex_r4/Register  init=1  rst=off  en=off  dbl_ovrd=off  block_type=register
      settings: xl_area=[1 1 0 0 0 0 0]  block_version=VER_STRING_GOES_HERE
  FFT tvalid : Outport
  FFT tdata_re : Outport  Port=2
  FFT tdata_im : Outport  Port=3
  FFT tlast : Outport  Port=4
  FIFO tready : Outport  Port=5
  wires:
  AXI FIFO/2 -> I/Q Slice/1(IQ)
  AXI FIFO/1 -> FFT tvalid/1, Gateway Out1/1, Logical2/1
  AXI FIFO/3 -> Logical2/2
  Gateway Out8/1 -> FIFO/1  "FFT ready"
  I/Q Slice/1(I) -> FFT tdata_re/1
  I/Q Slice/2(Q) -> FFT tdata_im/1, Gateway Out2/1
  FFT tready/1 -> Gateway Out8/1, AXI FIFO/1
  data tvalid/1 -> Gateway Out5/1, AXI FIFO/2
  data tlast/1 -> Gateway Out6/1, AXI FIFO/4
  AXI FIFO/4 -> FIFO tready/1, Gateway Out4/1
  Gateway Out1/1 -> FIFO/2  "FIFO out valid"
  Gateway Out2/1 -> FIFO/3  "Q out"
  Gateway Out3/1 -> FIFO/4  "tlast out"
  Gateway Out4/1 -> FIFO/5  "FIFO ready"
  Gateway Out5/1 -> FIFO/6  "valid in"
  Gateway Out6/1 -> FIFO/7  "tlast in"
  Logical2/1 -> FFT tlast/1, Gateway Out3/1
  I/1 -> I/Q Concat/1(I)
  Q/1 -> I/Q Concat/2(Q)
  I/Q Concat/1(IQ) -> AXI FIFO/3
  reset/1 -> Inverter/1
  Inverter/1 -> Register2/1
  ? -> FIFO/8  "Reset"
  Gateway Out9/1 -> FIFO/9  "FIFO Occ"
  AXI FIFO/5 -> Gateway Out9/1
  Register2/1 -> ?

== system wlan_phy_rx_pmd/CFO & Samp Buffer/Negedge ==
  D : Inport
  Delay : xbsIndex_r4/Delay  rst=off  infoeditControl=Selection of Reset will increase slice count due to use of real FFs and instead of SRLs  en=off  latency=1  dbl_ovrd=off  reg_retiming=off  block_type=delay
      settings: infoedit=Hardware notes: A delay line is a chain, each link of which is an SRL16 followed by a flip-flop. If register retiming is enabled, the delay line is a chain of flip-flops.  xl_area=[1 1 0 0 0 0 0]  block_version=VER_STRING_GOES_HERE
  Inverter : xbsIndex_r4/Inverter  en=off  latency=0  dbl_ovrd=off  block_type=inv
      settings: xl_area=[0 0 0 0 0 0 0]  block_version=VER_STRING_GOES_HERE
  Logical1 : xbsIndex_r4/Logical  logical_function=AND  inputs=2  en=off  latency=0  precision=Full  arith_type=Unsigned  n_bits=8  bin_pt=2  align_bp=on  dbl_ovrd=off  block_type=logical
      settings: xl_area=[1 0 0 1 0 0 0]  block_version=VER_STRING_GOES_HERE
  Q : Outport
  wires:
  D/1 -> Delay/1, Inverter/1
  Logical1/1 -> Q/1
  Delay/1 -> Logical1/1
  Inverter/1 -> Logical1/2

== system wlan_phy_rx_pmd/CFO & Samp Buffer/Samp Buffer ==
  note: Circular sample buffer records all incoming I/Q samples post pkt det
      | Samples are unloaded from the buffer after LTS sync and fed into
      | the FFT (via CFO correction and a FIFO)
      |
      | This buffer is required because 802.11 uses the same preamble samples
      | (the LTS) for sample-level synchronization and CFO estimation. The
      | received LTS samples must be saved and CFO-corrected before the
      | FFT to avoid degrading the channel estimates.
  Pkt Det : Inport
  Pkt Done : Inport  Port=2
  rx I : Inport  Port=3
  rx Q : Inport  Port=4
  IQ valid : Inport  Port=5
  LTS Sync 2 : Inport  Port=6
  FIFO tready : Inport  Port=7
  Circular Sample Buffer : xbsIndex_r4/Dual Port RAM  depth=2^n_bits_preFFT_sampBuff  initVector=1 * repmat([0:63], 1, 4)  distributed_mem=Block RAM  init_a=0  init_b=0  rst_a=off  rst_b=off  en_a=off  en_b=off  latency=1  write_mode_A=Read After Write  write_mode_B=Read After Write  dbl_ovrd=off  optimize=Area  block_type=dpram
  Concat : Subsystem
  Constant3 : xbsIndex_r4/Constant  const=0  gui_display_data_type=Fixed-point  arith_type=Unsigned  n_bits=32  bin_pt=0  preci_type=Single  exp_width=8  frac_width=24  explicit_period=off  period=8  equ=P=C  opselect=C  inp2=PCIN>>17  opr=+  inp1=P  carry=CIN  dbl_ovrd=off  block_type=constant
  Constant4 : xbsIndex_r4/Constant  const=0  gui_display_data_type=Boolean  arith_type=Boolean  n_bits=6  bin_pt=0  preci_type=Single  exp_width=8  frac_width=24  explicit_period=off  period=8  equ=P=C  opselect=C  inp2=PCIN>>17  opr=+  inp1=P  carry=CIN  dbl_ovrd=off  block_type=constant
  Counter : xbsIndex_r4/Counter  cnt_type=Free Running  cnt_to=Inf  operation=Up  start_count=0  cnt_by_val=1  arith_type=Unsigned  n_bits=n_bits_preFFT_sampBuff  bin_pt=0  load_pin=off  rst=off  en=on  explicit_period=off  period=8  dbl_ovrd=off  use_behavioral_HDL=off  implementation=Fabric  block_type=counter
  Delay : xbsIndex_r4/Delay  rst=off  infoeditControl=Selection of Reset will increase slice count due to use of real FFs and instead of SRLs  en=off  latency=1  dbl_ovrd=off  reg_retiming=off  block_type=delay
  Delay2 : xbsIndex_r4/Delay  rst=off  infoeditControl=Selection of Reset will increase slice count due to use of real FFs and instead of SRLs  en=off  latency=1  dbl_ovrd=off  reg_retiming=off  block_type=delay
  FFT Load Ctrl : Subsystem
  FSM : Subsystem
  Gateway Out{1..7} (7 alike) : xbsIndex_r4/Gateway Out  inherit_from_input=off  hdl_port=off  timing_constraint=None  locs_specified=off  LOCs={}  UseAsDAC=off  DACChannel='1'  block_type=gatewayout
  Logical2 : xbsIndex_r4/Logical  logical_function=AND  inputs=2  en=off  latency=0  precision=Full  arith_type=Unsigned  n_bits=16  bin_pt=0  align_bp=on  dbl_ovrd=off  block_type=logical
  Posedge : Subsystem
  Posedge3 : Subsystem
  Register : xbsIndex_r4/Register  init=0  rst=off  en=off  dbl_ovrd=off  block_type=register
  Register1 : xbsIndex_r4/Register  init=0  rst=off  en=off  dbl_ovrd=off  block_type=register
  S-R Latch : Subsystem
  Scope : Scope  NumInputPorts=7
      settings: TimeRange=10000  YMin=-0.25~-0.25~0~0~0~-0.1~-0.1  YMax=0.2~0.2~300~300~4000~1.1~1.1  SaveName=ScopeData25
  Slice : Subsystem
  Terminator : Terminator
  dbg_payload : xbsIndex_r4/Gateway Out  inherit_from_input=off  hdl_port=on  timing_constraint=None  locs_specified=off  LOCs={}  UseAsDAC=off  DACChannel='1'  block_type=gatewayout
  I : Outport
  Q : Outport  Port=2
  Buff Rd : Outport  Port=3
  " IQ valid" : Outport  Port=4
  tlast : Outport  Port=5
  tag scope: OFDM_RX_RUNNING_DSSS_BLOCK=global
  wires:
  Concat/1(IQ) -> Circular Sample Buffer/2
  Circular Sample Buffer/1 -> Terminator/1
  FFT Load Ctrl/1(Rd Addr) -> Circular Sample Buffer/4, Gateway Out1/1
  Constant3/1 -> Circular Sample Buffer/5
  Constant4/1 -> Circular Sample Buffer/6
  Logical2/1 -> Posedge/1(D)
  Posedge/1(Q) -> FFT Load Ctrl/3(LTS Sync), FSM/2(LTS)
  FFT Load Ctrl/5(FFT Load Done) -> FSM/1(FFT Load Done), Gateway Out7/1
  FSM/2(FFT Data load) -> FFT Load Ctrl/5(FFT Load)
  Circular Sample Buffer/2 -> Slice/1(IQ)
  FSM/1(FFT skip sel) -> FFT Load Ctrl/4(Skip Sel)
  FFT Load Ctrl/3(FFT tvalid) -> Delay/1, Posedge3/1(D)
  FFT Load Ctrl/2(Reading) -> Delay2/1
  Pkt Done/1 -> FSM/3(Pkt Done), S-R Latch/2(R), Gateway Out4/1
  rx I/1 -> Concat/1(I), Gateway Out6/1
  rx Q/1 -> Concat/2(Q)
  IQ valid/1 -> Circular Sample Buffer/3, Counter/1
  Slice/1(I) -> I/1, Gateway Out5/1
  Slice/2(Q) -> Q/1
  FIFO tready/1 -> FFT Load Ctrl/2(data tready)
  Delay2/1 -> Buff Rd/1
  Delay/1 -> " IQ valid"/1
  FFT Load Ctrl/4(FFT tlast) -> tlast/1
  LTS Sync 2/1 -> Logical2/1
  Pkt Det/1 -> Logical2/2, Gateway Out3/1
  Gateway Out5/1 -> Scope/2  "FFT In I"
  Gateway Out1/1 -> Scope/3  "Rd Addr"
  Gateway Out2/1 -> Scope/4  "Wr Addr"
  Gateway Out3/1 -> Scope/5  "Pkt Det"
  Posedge3/1(Q) -> S-R Latch/1(S)
  S-R Latch/1(Q) -> Register/1, [OFDM_RX_RUNNING_DSSS_BLOCK]
  Gateway Out4/1 -> Scope/6  "Pkt Done"
  Gateway Out6/1 -> Scope/1  "ADC I"
  Register/1 -> Register1/1
  Register1/1 -> dbg_payload/1
  Gateway Out7/1 -> Scope/7  "FFT Load Done"
  Counter/1 -> Circular Sample Buffer/1, FFT Load Ctrl/1(Wr Addr), Gateway Out2/1
  FSM/3(FFT LTS load) -> FFT Load Ctrl/6(FFT LTS Load)
